simulink model "AcasXuClosedLoop_all_comp"  (Simulink 10.0)
settings: Solver=VariableStepAuto  StartTime=0.0  StopTime=20  RelTol=1e-3
libraries: neural x14040, simulink x2
(part 31 of 534)

== system AcasXuClosedLoop_all_comp/networks/Networks_m_7/AcasXu_4_7/Layer 5 ==
  "a{4} " : Inport  PortDimensions=50
      mask Display: e = 0.06;c1 = [120 230 180]/255;c2 = ([120 230 180]+0)/(256+128);c3 = ([120 230 180]+128)/(256+128);patch([0 1 1 0],[0 0 1 1],c1);patch([0 1 1 1-e 1-e 0+e],[0 0 1 1-e 0+e 0+e],c2);patch([0 0 1 1-e 0+e 0+e],[0 1 1 1-e 1-e 0+e],c3);plot([0 1 1 0 0],[0 0 1 1 0]);
  Delays 1 : Subsystem
      mask Display: e = 0.06;c1 = [120 150 230]/255;c2 = ([120 150 230]+0)/383;c3 = ([120 150 230]+128)/383;patch([0 1 1 0],[0 0 1 1],c1);patch([0 1 1 1-e 1-e 0+e],[0 0 1 1-e 0+e 0+e],c2);patch([0 0 1 1-e 0+e 0+e],[0 1 1 1-e 1-e 0+e],c3);plot([0 1 1 0 0],[0 0 1 1 0]);disp('0');
  "LW{5,4}" : Subsystem
      mask Display: e = 0.06;c1 = [120 150 230]/255;c2 = ([120 150 230]+0)/383;c3 = ([120 150 230]+128)/383;patch([0 1 1 0],[0 0 1 1],c1);patch([0 1 1 1-e 1-e 0+e],[0 0 1 1-e 0+e 0+e],c2);patch([0 0 1 1-e 0+e 0+e],[0 1 1 1-e 1-e 0+e],c3);plot([0 1 1 0 0],[0 0 1 1 0]);disp('W');
  "b{5}" : Constant  Value=[-1.29535000000000000142108547152020037174224853515625;4.1142500000000001847411112976260483264923095703125;0.037726299999999997336619372845234465785324573516845703125;-0.0252420999999999999541255846224885317496955394744873046875;-3.103639999999999954383156364201568067073822021484375;-0.0961853000000000013525180975193507038056850433349609375;2.682910000000000128039800983970053493976593017578125;2.18447000000000013386625141720287501811981201171875;-0.540381000000000000227373675443232059478759765625;-0.8092319999999999513562443098635412752628326416015625;5.54617999999999966576069709844887256622314453125;-1.3036700000000001065103560904390178620815277099609375;-0.173094999999999998863131622783839702606201171875;-1.215829999999999966320274324971251189708709716796875;0.5644869999999999610196255162009038031101226806640625;0.117124000000000005883293852093629539012908935546875;0.0181935000000000013375967000683885999023914337158203125;-2.12652000000000018786749933497048914432525634765625;-1.331609999999999960351715344586409628391265869140625;1.316429999999999989057641869294457137584686279296875;-0.919273000000000006792788553866557776927947998046875;-0.2169830000000000091997520712538971565663814544677734375;0.266203000000000022939872224014834500849246978759765625;3.121090000000000141966438604868017137050628662109375;-0.8987920000000000353423956767073832452297210693359375;-1.9803100000000000147082346302340738475322723388671875;-1.33654999999999990478727340814657509326934814453125;0.5149690000000000100754959930782206356525421142578125;-0.01195290000000000067703620487691296148113906383514404296875;-5.355109999999999814690454513765871524810791015625;1.3112399999999999611333123539225198328495025634765625;0.048415300000000001390265680356606026180088520050048828125;1.996750000000000024868995751603506505489349365234375;0.14216200000000001057998133546789176762104034423828125;-0.06109789999999999665636352119690855033695697784423828125;-2.012399999999999966604491419275291264057159423828125;1.7953900000000000414246414948138408362865447998046875;0.1728530000000000066417982225175364874303340911865234375;-0.411121000000000014207302001523203216493129730224609375;0.1690629999999999910631487409773399122059345245361328125;2.4055499999999998550492819049395620822906494140625;-0.06303730000000000444071446281668613664805889129638671875;1.6415100000000000246558329308754764497280120849609375;1.31810999999999989285015544737689197063446044921875;3.186749999999999971578290569595992565155029296875;2.004440000000000221547225009999237954616546630859375;-0.82457700000000000439825953435502015054225921630859375;-0.762615999999999960579089020029641687870025634765625;-1.0003800000000000469668748337426222860813140869140625;2.399080000000000101323394119390286505222320556640625]
      mask Display: e = 0.06;c1 = [120 150 230]/255;c2 = ([120 150 230]+0)/383;c3 = ([120 150 230]+128)/383;patch([0 1 1 0],[0 0 1 1],c1);patch([0 1 1 1-e 1-e 0+e],[0 0 1 1-e 0+e 0+e],c2);patch([0 0 1 1-e 0+e 0+e],[0 1 1 1-e 1-e 0+e],c3);plot([0 1 1 0 0],[0 0 1 1 0]);disp('b');
  netsum : Sum  Inputs=2  SaturateOnIntegerOverflow=on  InputSameDT=on  OutDataTypeStr=Inherit: Same as first input
      mask Display: e = 0.06;c1 = [120 150 230]/255;c2 = ([120 150 230]+0)/383;c3 = ([120 150 230]+128)/383;patch([0 1 1 0],[0 0 1 1],c1);patch([0 1 1 1-e 1-e 0+e],[0 0 1 1-e 0+e 0+e],c2);patch([0 0 1 1-e 0+e 0+e],[0 1 1 1-e 1-e 0+e],c3);plot([0 1 1 0 0],[0 0 1 1 0]);disp('+');
  poslin : neural/Transfer Functions/poslin
      settings: SourceProductName=Deep Learning Toolbox  SourceProductBaseCode=NN
  "a{5}" : Outport  InitialOutput=[0;0;0;0;0;0;0;0;0;0;0;0;0;0;0;0;0;0;0;0;0;0;0;0;0;0;0;0;0;0;0;0;0;0;0;0;0;0;0;0;0;0;0;0;0;0;0;0;0;0]
      mask Display: e = 0.06;c1 = [120 230 180]/255;c2 = ([120 230 180]+0)/(256+128);c3 = ([120 230 180]+128)/(256+128);patch([0 1 1 0],[0 0 1 1],c1);patch([0 1 1 1-e 1-e 0+e],[0 0 1 1-e 0+e 0+e],c2);patch([0 0 1 1-e 0+e 0+e],[0 1 1 1-e 1-e 0+e],c3);plot([0 1 1 0 0],[0 0 1 1 0]);
  wires:
  poslin/1 -> "a{5}"/1
  netsum/1 -> poslin/1
  "b{5}"/1 -> netsum/2
  "LW{5,4}"/1("lz{5,4}") -> netsum/1
  Delays 1/1("pd{5,4}") -> "LW{5,4}"/1("ad{5,4}")
  "a{4} "/1 -> Delays 1/1("p{5}")

== system AcasXuClosedLoop_all_comp/networks/Networks_m_7/AcasXu_4_7/Layer 6 ==
  "a{5} " : Inport  PortDimensions=50
      mask Display: e = 0.06;c1 = [120 230 180]/255;c2 = ([120 230 180]+0)/(256+128);c3 = ([120 230 180]+128)/(256+128);patch([0 1 1 0],[0 0 1 1],c1);patch([0 1 1 1-e 1-e 0+e],[0 0 1 1-e 0+e 0+e],c2);patch([0 0 1 1-e 0+e 0+e],[0 1 1 1-e 1-e 0+e],c3);plot([0 1 1 0 0],[0 0 1 1 0]);
  Delays 1 : Subsystem
      mask Display: e = 0.06;c1 = [120 150 230]/255;c2 = ([120 150 230]+0)/383;c3 = ([120 150 230]+128)/383;patch([0 1 1 0],[0 0 1 1],c1);patch([0 1 1 1-e 1-e 0+e],[0 0 1 1-e 0+e 0+e],c2);patch([0 0 1 1-e 0+e 0+e],[0 1 1 1-e 1-e 0+e],c3);plot([0 1 1 0 0],[0 0 1 1 0]);disp('0');
  "LW{6,5}" : Subsystem
      mask Display: e = 0.06;c1 = [120 150 230]/255;c2 = ([120 150 230]+0)/383;c3 = ([120 150 230]+128)/383;patch([0 1 1 0],[0 0 1 1],c1);patch([0 1 1 1-e 1-e 0+e],[0 0 1 1-e 0+e 0+e],c2);patch([0 0 1 1-e 0+e 0+e],[0 1 1 1-e 1-e 0+e],c3);plot([0 1 1 0 0],[0 0 1 1 0]);disp('W');
  "b{6}" : Constant  Value=[3.367739999999999955804241835721768438816070556640625;2.545879999999999920845539236324839293956756591796875;-0.0389668999999999987604581974665052257478237152099609375;-1.9147499999999999520383653361932374536991119384765625;-2.70427000000000017365664461976848542690277099609375;-3.99943999999999988403942552395164966583251953125;-0.4635569999999999968309793985099531710147857666015625;1.5156099999999999017319396443781442940235137939453125;0.5237119999999999553352836301201023161411285400390625;2.05891000000000001790567694115452468395233154296875;-4.25011999999999989796606314484961330890655517578125;0.775074000000000040699887904338538646697998046875;0.5163379999999999636628444932284764945507049560546875;-0.03237210000000000087450047203674330376088619232177734375;-3.05037000000000002586375558166764676570892333984375;-5.9134299999999999641886461176909506320953369140625;-0.05554019999999999790407656519164447672665119171142578125;7.9652300000000000324007487506605684757232666015625;-0.28509499999999998731681216668221168220043182373046875;4.55646000000000039875658330856822431087493896484375;-3.091439999999999965751840136363171041011810302734375;-0.01152899999999999931798999597276633721776306629180908203125;0.88857500000000000373034936274052597582340240478515625;1.3019700000000000716937620381941087543964385986328125;-1.7894799999999999595701183352502994239330291748046875;-2.40425000000000022026824808563105762004852294921875;-0.036211300000000001819699946281616576015949249267578125;-2.94575999999999993406163412146270275115966796875;-5.16894999999999971151964928139932453632354736328125;-4.540919999999999845385900698602199554443359375;0.95291599999999998527044908769312314689159393310546875;-0.0280836999999999996358024390019636484794318675994873046875;0.6533210000000000405862010666169226169586181640625;1.7611399999999999277378037731978110969066619873046875;-0.2219969999999999998863131622783839702606201171875;1.140579999999999927240423858165740966796875;-3.731050000000000199662508748588152229785919189453125;0.499856999999999995765165294869802892208099365234375;0.9889639999999999542268369623343460261821746826171875;-3.509069999999999911466375124291516840457916259765625;-3.913670000000000204209982257452793419361114501953125;-2.9911799999999999499777914024889469146728515625;4.86252999999999957481122692115604877471923828125;-2.406969999999999831885588719160296022891998291015625;0.58633900000000005459099838844849728047847747802734375;-0.031272000000000001185274101089817122556269168853759765625;-4.6410099999999996356336851022206246852874755859375;-5.66669999999999962625452099018730223178863525390625;-2.46628999999999987124965628026984632015228271484375;1.76560000000000005826450433232821524143218994140625]
      mask Display: e = 0.06;c1 = [120 150 230]/255;c2 = ([120 150 230]+0)/383;c3 = ([120 150 230]+128)/383;patch([0 1 1 0],[0 0 1 1],c1);patch([0 1 1 1-e 1-e 0+e],[0 0 1 1-e 0+e 0+e],c2);patch([0 0 1 1-e 0+e 0+e],[0 1 1 1-e 1-e 0+e],c3);plot([0 1 1 0 0],[0 0 1 1 0]);disp('b');
  netsum : Sum  Inputs=2  SaturateOnIntegerOverflow=on  InputSameDT=on  OutDataTypeStr=Inherit: Same as first input
      mask Display: e = 0.06;c1 = [120 150 230]/255;c2 = ([120 150 230]+0)/383;c3 = ([120 150 230]+128)/383;patch([0 1 1 0],[0 0 1 1],c1);patch([0 1 1 1-e 1-e 0+e],[0 0 1 1-e 0+e 0+e],c2);patch([0 0 1 1-e 0+e 0+e],[0 1 1 1-e 1-e 0+e],c3);plot([0 1 1 0 0],[0 0 1 1 0]);disp('+');
  poslin : neural/Transfer Functions/poslin
      settings: SourceProductName=Deep Learning Toolbox  SourceProductBaseCode=NN
  "a{6}" : Outport  InitialOutput=[0;0;0;0;0;0;0;0;0;0;0;0;0;0;0;0;0;0;0;0;0;0;0;0;0;0;0;0;0;0;0;0;0;0;0;0;0;0;0;0;0;0;0;0;0;0;0;0;0;0]
      mask Display: e = 0.06;c1 = [120 230 180]/255;c2 = ([120 230 180]+0)/(256+128);c3 = ([120 230 180]+128)/(256+128);patch([0 1 1 0],[0 0 1 1],c1);patch([0 1 1 1-e 1-e 0+e],[0 0 1 1-e 0+e 0+e],c2);patch([0 0 1 1-e 0+e 0+e],[0 1 1 1-e 1-e 0+e],c3);plot([0 1 1 0 0],[0 0 1 1 0]);
  wires:
  poslin/1 -> "a{6}"/1
  netsum/1 -> poslin/1
  "b{6}"/1 -> netsum/2
  "LW{6,5}"/1("lz{6,5}") -> netsum/1
  Delays 1/1("pd{6,5}") -> "LW{6,5}"/1("ad{6,5}")
  "a{5} "/1 -> Delays 1/1("p{6}")

== system AcasXuClosedLoop_all_comp/networks/Networks_m_7/AcasXu_4_7/Layer 7 ==
  "a{6} " : Inport  PortDimensions=50
      mask Display: e = 0.06;c1 = [120 230 180]/255;c2 = ([120 230 180]+0)/(256+128);c3 = ([120 230 180]+128)/(256+128);patch([0 1 1 0],[0 0 1 1],c1);patch([0 1 1 1-e 1-e 0+e],[0 0 1 1-e 0+e 0+e],c2);patch([0 0 1 1-e 0+e 0+e],[0 1 1 1-e 1-e 0+e],c3);plot([0 1 1 0 0],[0 0 1 1 0]);
  Delays 1 : Subsystem
      mask Display: e = 0.06;c1 = [120 150 230]/255;c2 = ([120 150 230]+0)/383;c3 = ([120 150 230]+128)/383;patch([0 1 1 0],[0 0 1 1],c1);patch([0 1 1 1-e 1-e 0+e],[0 0 1 1-e 0+e 0+e],c2);patch([0 0 1 1-e 0+e 0+e],[0 1 1 1-e 1-e 0+e],c3);plot([0 1 1 0 0],[0 0 1 1 0]);disp('0');
  "LW{7,6}" : Subsystem
      mask Display: e = 0.06;c1 = [120 150 230]/255;c2 = ([120 150 230]+0)/383;c3 = ([120 150 230]+128)/383;patch([0 1 1 0],[0 0 1 1],c1);patch([0 1 1 1-e 1-e 0+e],[0 0 1 1-e 0+e 0+e],c2);patch([0 0 1 1-e 0+e 0+e],[0 1 1 1-e 1-e 0+e],c3);plot([0 1 1 0 0],[0 0 1 1 0]);disp('W');
  "b{7}" : Constant  Value=[-0.0211303000000000011648904063576992484740912914276123046875;-0.0184695999999999992124966041728839627467095851898193359375;0.0182207999999999988027798991652161930687725543975830078125;-0.01842080000000000106208375427740975283086299896240234375;0.0182178999999999986780796490393186104483902454376220703125]
      mask Display: e = 0.06;c1 = [120 150 230]/255;c2 = ([120 150 230]+0)/383;c3 = ([120 150 230]+128)/383;patch([0 1 1 0],[0 0 1 1],c1);patch([0 1 1 1-e 1-e 0+e],[0 0 1 1-e 0+e 0+e],c2);patch([0 0 1 1-e 0+e 0+e],[0 1 1 1-e 1-e 0+e],c3);plot([0 1 1 0 0],[0 0 1 1 0]);disp('b');
  netsum : Sum  Inputs=2  SaturateOnIntegerOverflow=on  InputSameDT=on  OutDataTypeStr=Inherit: Same as first input
      mask Display: e = 0.06;c1 = [120 150 230]/255;c2 = ([120 150 230]+0)/383;c3 = ([120 150 230]+128)/383;patch([0 1 1 0],[0 0 1 1],c1);patch([0 1 1 1-e 1-e 0+e],[0 0 1 1-e 0+e 0+e],c2);patch([0 0 1 1-e 0+e 0+e],[0 1 1 1-e 1-e 0+e],c3);plot([0 1 1 0 0],[0 0 1 1 0]);disp('+');
  purelin : neural/Transfer Functions/purelin
      settings: SourceProductName=Deep Learning Toolbox
  "a{7}" : Outport  InitialOutput=[0;0;0;0;0]
      mask Display: e = 0.06;c1 = [120 230 180]/255;c2 = ([120 230 180]+0)/(256+128);c3 = ([120 230 180]+128)/(256+128);patch([0 1 1 0],[0 0 1 1],c1);patch([0 1 1 1-e 1-e 0+e],[0 0 1 1-e 0+e 0+e],c2);patch([0 0 1 1-e 0+e 0+e],[0 1 1 1-e 1-e 0+e],c3);plot([0 1 1 0 0],[0 0 1 1 0]);
  wires:
  purelin/1 -> "a{7}"/1
  netsum/1 -> purelin/1
  "b{7}"/1 -> netsum/2
  "LW{7,6}"/1("lz{7,6}") -> netsum/1
  Delays 1/1("pd{7,6}") -> "LW{7,6}"/1("ad{7,6}")
  "a{6} "/1 -> Delays 1/1("p{7}")

== system AcasXuClosedLoop_all_comp/networks/Networks_m_7/AcasXu_4_7/Process Input 1 ==
  x : Inport  PortDimensions=5
      mask Display: e = 0.06;c1 = [120 230 180]/255;c2 = ([120 230 180]+0)/(256+128);c3 = ([120 230 180]+128)/(256+128);patch([0 1 1 0],[0 0 1 1],c1);patch([0 1 1 1-e 1-e 0+e],[0 0 1 1-e 0+e 0+e],c2);patch([0 0 1 1-e 0+e 0+e],[0 1 1 1-e 1-e 0+e],c3);plot([0 1 1 0 0],[0 0 1 1 0]);
  p : Outport  PortDimensions=5
      mask Display: e = 0.06;c1 = [120 230 180]/255;c2 = ([120 230 180]+0)/(256+128);c3 = ([120 230 180]+128)/(256+128);patch([0 1 1 0],[0 0 1 1],c1);patch([0 1 1 1-e 1-e 0+e],[0 0 1 1-e 0+e 0+e],c2);patch([0 0 1 1-e 0+e 0+e],[0 1 1 1-e 1-e 0+e],c3);plot([0 1 1 0 0],[0 0 1 1 0]);
  wires:
  x/1 -> p/1

== system AcasXuClosedLoop_all_comp/networks/Networks_m_7/AcasXu_4_7/Process Output 1 ==
  a : Inport  PortDimensions=5
      mask Display: e = 0.06;c1 = [120 230 180]/255;c2 = ([120 230 180]+0)/(256+128);c3 = ([120 230 180]+128)/(256+128);patch([0 1 1 0],[0 0 1 1],c1);patch([0 1 1 1-e 1-e 0+e],[0 0 1 1-e 0+e 0+e],c2);patch([0 0 1 1-e 0+e 0+e],[0 1 1 1-e 1-e 0+e],c3);plot([0 1 1 0 0],[0 0 1 1 0]);
  y : Outport  PortDimensions=5
      mask Display: e = 0.06;c1 = [120 230 180]/255;c2 = ([120 230 180]+0)/(256+128);c3 = ([120 230 180]+128)/(256+128);patch([0 1 1 0],[0 0 1 1],c1);patch([0 1 1 1-e 1-e 0+e],[0 0 1 1-e 0+e 0+e],c2);patch([0 0 1 1-e 0+e 0+e],[0 1 1 1-e 1-e 0+e],c3);plot([0 1 1 0 0],[0 0 1 1 0]);
  wires:
  a/1 -> y/1

== system AcasXuClosedLoop_all_comp/networks/Networks_m_7/AcasXu_5_7/Layer 1 ==
  "p{1}" : Inport  PortDimensions=5
      mask Display: e = 0.06;c1 = [120 230 180]/255;c2 = ([120 230 180]+0)/(256+128);c3 = ([120 230 180]+128)/(256+128);patch([0 1 1 0],[0 0 1 1],c1);patch([0 1 1 1-e 1-e 0+e],[0 0 1 1-e 0+e 0+e],c2);patch([0 0 1 1-e 0+e 0+e],[0 1 1 1-e 1-e 0+e],c3);plot([0 1 1 0 0],[0 0 1 1 0]);
  Delays 1 : Subsystem
      mask Display: e = 0.06;c1 = [120 150 230]/255;c2 = ([120 150 230]+0)/383;c3 = ([120 150 230]+128)/383;patch([0 1 1 0],[0 0 1 1],c1);patch([0 1 1 1-e 1-e 0+e],[0 0 1 1-e 0+e 0+e],c2);patch([0 0 1 1-e 0+e 0+e],[0 1 1 1-e 1-e 0+e],c3);plot([0 1 1 0 0],[0 0 1 1 0]);disp('0');
  "IW{1,1}" : Subsystem
      mask Display: e = 0.06;c1 = [120 150 230]/255;c2 = ([120 150 230]+0)/383;c3 = ([120 150 230]+128)/383;patch([0 1 1 0],[0 0 1 1],c1);patch([0 1 1 1-e 1-e 0+e],[0 0 1 1-e 0+e 0+e],c2);patch([0 0 1 1-e 0+e 0+e],[0 1 1 1-e 1-e 0+e],c3);plot([0 1 1 0 0],[0 0 1 1 0]);disp('W');
  "b{1}" : Constant  Value=[0.025853700000000000291944246555431163869798183441162109375;-0.1536579999999999890381019440610543824732303619384765625;-0.09579020000000000589768234249277156777679920196533203125;0.01953850000000000031175062531474395655095577239990234375;-0.000513157999999999995220656412442394866957329213619232177734375;-0.256688000000000027256419343757443130016326904296875;-0.02045829999999999870841094207207788713276386260986328125;0.015036199999999999565858388450578786432743072509765625;-0.1940860000000000085140783312454004772007465362548828125;-0.6372079999999999966320274324971251189708709716796875;-0.398884999999999989572785352720529772341251373291015625;0.0055165999999999999758859559051415999419987201690673828125;-0.21925200000000000244426701101474463939666748046875;-0.322757000000000016104451106002670712769031524658203125;0.19237599999999999145217088880599476397037506103515625;-0.0266212000000000011123990617534218472428619861602783203125;-0.1476059999999999872155598268363974057137966156005859375;0.159337000000000006405542762877303175628185272216796875;0.371367999999999975901943116696202196180820465087890625;-0.67358499999999998930633182681049220263957977294921875;0.0638948000000000015941026276777847670018672943115234375;-0.14384099999999999663913285985472612082958221435546875;-0.2489180000000000003712585794346523471176624298095703125;-0.592006000000000032201796784647740423679351806640625;0.127653999999999989700683045157347805798053741455078125;-0.253425999999999984613197057115030474960803985595703125;-0.5876360000000000471942485091858543455600738525390625;0.029080000000000001680877659282487002201378345489501953125;0.291185000000000027142732506035827100276947021484375;-0.419733999999999995988986256634234450757503509521484375;-0.1534250000000000058175686490358202718198299407958984375;0.12184399999999999397726213601345079950988292694091796875;0.30686299999999999688071739001316018402576446533203125;-0.286256000000000010441425501994672231376171112060546875;0.08300100000000000532995869662045151926577091217041015625;0.06258149999999999824051855057405191473662853240966796875;-0.41020299999999998430411096705938689410686492919921875;0.08292960000000000608810779567647841759026050567626953125;-0.36475800000000002665245801836135797202587127685546875;-0.210280999999999995697663734972593374550342559814453125;0.0819209999999999938236072694053291343152523040771484375;-0.38086900000000001309530262005864642560482025146484375;-0.36825099999999999500488456760649569332599639892578125;-0.060613199999999999023625463223652332089841365814208984375;0.08224700000000000066346927951599354855716228485107421875;-0.1641789999999999916102666475126170553267002105712890625;-0.10791199999999999403765826855305931530892848968505859375;-0.274878000000000011215917083973181433975696563720703125;0.520432000000000005712763595511205494403839111328125;0.027086800000000001154010220716372714377939701080322265625]
      mask Display: e = 0.06;c1 = [120 150 230]/255;c2 = ([120 150 230]+0)/383;c3 = ([120 150 230]+128)/383;patch([0 1 1 0],[0 0 1 1],c1);patch([0 1 1 1-e 1-e 0+e],[0 0 1 1-e 0+e 0+e],c2);patch([0 0 1 1-e 0+e 0+e],[0 1 1 1-e 1-e 0+e],c3);plot([0 1 1 0 0],[0 0 1 1 0]);disp('b');
  netsum : Sum  Inputs=2  SaturateOnIntegerOverflow=on  InputSameDT=on  OutDataTypeStr=Inherit: Same as first input
      mask Display: e = 0.06;c1 = [120 150 230]/255;c2 = ([120 150 230]+0)/383;c3 = ([120 150 230]+128)/383;patch([0 1 1 0],[0 0 1 1],c1);patch([0 1 1 1-e 1-e 0+e],[0 0 1 1-e 0+e 0+e],c2);patch([0 0 1 1-e 0+e 0+e],[0 1 1 1-e 1-e 0+e],c3);plot([0 1 1 0 0],[0 0 1 1 0]);disp('+');
  poslin : neural/Transfer Functions/poslin
      settings: SourceProductName=Deep Learning Toolbox  SourceProductBaseCode=NN
  "a{1}" : Outport  InitialOutput=[0;0;0;0;0;0;0;0;0;0;0;0;0;0;0;0;0;0;0;0;0;0;0;0;0;0;0;0;0;0;0;0;0;0;0;0;0;0;0;0;0;0;0;0;0;0;0;0;0;0]
      mask Display: e = 0.06;c1 = [120 230 180]/255;c2 = ([120 230 180]+0)/(256+128);c3 = ([120 230 180]+128)/(256+128);patch([0 1 1 0],[0 0 1 1],c1);patch([0 1 1 1-e 1-e 0+e],[0 0 1 1-e 0+e 0+e],c2);patch([0 0 1 1-e 0+e 0+e],[0 1 1 1-e 1-e 0+e],c3);plot([0 1 1 0 0],[0 0 1 1 0]);
  wires:
  poslin/1 -> "a{1}"/1
  netsum/1 -> poslin/1
  "b{1}"/1 -> netsum/2
  "IW{1,1}"/1("iz{1,1}") -> netsum/1
  Delays 1/1("pd{1,1}") -> "IW{1,1}"/1("pd{1,1}")
  "p{1}"/1 -> Delays 1/1("p{1}")

== system AcasXuClosedLoop_all_comp/networks/Networks_m_7/AcasXu_5_7/Layer 2 ==
  "a{1} " : Inport  PortDimensions=50
      mask Display: e = 0.06;c1 = [120 230 180]/255;c2 = ([120 230 180]+0)/(256+128);c3 = ([120 230 180]+128)/(256+128);patch([0 1 1 0],[0 0 1 1],c1);patch([0 1 1 1-e 1-e 0+e],[0 0 1 1-e 0+e 0+e],c2);patch([0 0 1 1-e 0+e 0+e],[0 1 1 1-e 1-e 0+e],c3);plot([0 1 1 0 0],[0 0 1 1 0]);
  Delays 1 : Subsystem
      mask Display: e = 0.06;c1 = [120 150 230]/255;c2 = ([120 150 230]+0)/383;c3 = ([120 150 230]+128)/383;patch([0 1 1 0],[0 0 1 1],c1);patch([0 1 1 1-e 1-e 0+e],[0 0 1 1-e 0+e 0+e],c2);patch([0 0 1 1-e 0+e 0+e],[0 1 1 1-e 1-e 0+e],c3);plot([0 1 1 0 0],[0 0 1 1 0]);disp('0');
  "LW{2,1}" : Subsystem
      mask Display: e = 0.06;c1 = [120 150 230]/255;c2 = ([120 150 230]+0)/383;c3 = ([120 150 230]+128)/383;patch([0 1 1 0],[0 0 1 1],c1);patch([0 1 1 1-e 1-e 0+e],[0 0 1 1-e 0+e 0+e],c2);patch([0 0 1 1-e 0+e 0+e],[0 1 1 1-e 1-e 0+e],c3);plot([0 1 1 0 0],[0 0 1 1 0]);disp('W');
  "b{2}" : Constant  Value=[0.11085000000000000408562073062057606875896453857421875;-0.11289599999999999635491576555068604648113250732421875;-0.58576700000000003765165956792770884931087493896484375;-0.196506999999999987238652465748600661754608154296875;-0.0165311999999999993671284670426757656969130039215087890625;-0.039218099999999998794830702308900072239339351654052734375;-0.0174786000000000003862243858065994572825729846954345703125;-1.5495699999999998919264498908887617290019989013671875;0.135572999999999999065636302475468255579471588134765625;1.124940000000000051016968427575193345546722412109375;-0.0162874999999999998945288126606101286597549915313720703125;1.3120499999999999385380533567513339221477508544921875;1.809779999999999944293449516408145427703857421875;-0.8840179999999999704840547565254382789134979248046875;0.29213699999999998002664369778358377516269683837890625;-0.2073230000000000072812866847016266547143459320068359375;-0.0147159000000000005081712828314266516827046871185302734375;0.2425299999999999955857532540903775952756404876708984375;0.325168999999999985828225135264801792800426483154296875;0.4723910000000000053432813729159533977508544921875;0.804680999999999979621634338400326669216156005859375;-0.00365592999999999994364952016212555463425815105438232421875;-0.032890700000000001879119082559554954059422016143798828125;0.3019439999999999901802993917954154312610626220703125;0.90261599999999997390176531553152017295360565185546875;0.19002900000000000346744855050928890705108642578125;-0.047453900000000000247712961254364927299320697784423828125;-1.1688199999999999700861508244997821748256683349609375;-0.00785373999999999967969177561144533683545887470245361328125;-0.054647099999999997066435497572456370107829570770263671875;0.303854000000000012970957641300628893077373504638671875;-0.01024830000000000011783907183371411520056426525115966796875;-0.1605100000000000137756472895489423535764217376708984375;0.0523083000000000020168755554550443775951862335205078125;-0.3190589999999999815116780155221931636333465576171875;0.469619999999999981898923806511447764933109283447265625;0.2776680000000000259063881458132527768611907958984375;-0.86825399999999997024957565372460521757602691650390625;0.287171000000000009588774219082552008330821990966796875;1.2200699999999999878497192185022868216037750244140625;-0.09989620000000000443751702050576568581163883209228515625;0.84266300000000005088196530778077431023120880126953125;1.410579999999999945003992252168245613574981689453125;-0.0578737000000000001431743612556601874530315399169921875;-0.442159000000000024233060003098216839134693145751953125;-1.380290000000000016910917111090384423732757568359375;-0.56972100000000003294786665719584561884403228759765625;0.12313899999999999845812226340058259665966033935546875;0.63547600000000004083489102413295768201351165771484375;0.1860570000000000001616484723854227922856807708740234375]
      mask Display: e = 0.06;c1 = [120 150 230]/255;c2 = ([120 150 230]+0)/383;c3 = ([120 150 230]+128)/383;patch([0 1 1 0],[0 0 1 1],c1);patch([0 1 1 1-e 1-e 0+e],[0 0 1 1-e 0+e 0+e],c2);patch([0 0 1 1-e 0+e 0+e],[0 1 1 1-e 1-e 0+e],c3);plot([0 1 1 0 0],[0 0 1 1 0]);disp('b');
  netsum : Sum  Inputs=2  SaturateOnIntegerOverflow=on  InputSameDT=on  OutDataTypeStr=Inherit: Same as first input
      mask Display: e = 0.06;c1 = [120 150 230]/255;c2 = ([120 150 230]+0)/383;c3 = ([120 150 230]+128)/383;patch([0 1 1 0],[0 0 1 1],c1);patch([0 1 1 1-e 1-e 0+e],[0 0 1 1-e 0+e 0+e],c2);patch([0 0 1 1-e 0+e 0+e],[0 1 1 1-e 1-e 0+e],c3);plot([0 1 1 0 0],[0 0 1 1 0]);disp('+');
  poslin : neural/Transfer Functions/poslin
      settings: SourceProductName=Deep Learning Toolbox  SourceProductBaseCode=NN
  "a{2}" : Outport  InitialOutput=[0;0;0;0;0;0;0;0;0;0;0;0;0;0;0;0;0;0;0;0;0;0;0;0;0;0;0;0;0;0;0;0;0;0;0;0;0;0;0;0;0;0;0;0;0;0;0;0;0;0]
      mask Display: e = 0.06;c1 = [120 230 180]/255;c2 = ([120 230 180]+0)/(256+128);c3 = ([120 230 180]+128)/(256+128);patch([0 1 1 0],[0 0 1 1],c1);patch([0 1 1 1-e 1-e 0+e],[0 0 1 1-e 0+e 0+e],c2);patch([0 0 1 1-e 0+e 0+e],[0 1 1 1-e 1-e 0+e],c3);plot([0 1 1 0 0],[0 0 1 1 0]);
  wires:
  poslin/1 -> "a{2}"/1
  netsum/1 -> poslin/1
  "b{2}"/1 -> netsum/2
  "LW{2,1}"/1("lz{2,1}") -> netsum/1
  Delays 1/1("pd{2,1}") -> "LW{2,1}"/1("ad{2,1}")
  "a{1} "/1 -> Delays 1/1("p{2}")

== system AcasXuClosedLoop_all_comp/networks/Networks_m_7/AcasXu_5_7/Layer 3 ==
  "a{2} " : Inport  PortDimensions=50
      mask Display: e = 0.06;c1 = [120 230 180]/255;c2 = ([120 230 180]+0)/(256+128);c3 = ([120 230 180]+128)/(256+128);patch([0 1 1 0],[0 0 1 1],c1);patch([0 1 1 1-e 1-e 0+e],[0 0 1 1-e 0+e 0+e],c2);patch([0 0 1 1-e 0+e 0+e],[0 1 1 1-e 1-e 0+e],c3);plot([0 1 1 0 0],[0 0 1 1 0]);
  Delays 1 : Subsystem
      mask Display: e = 0.06;c1 = [120 150 230]/255;c2 = ([120 150 230]+0)/383;c3 = ([120 150 230]+128)/383;patch([0 1 1 0],[0 0 1 1],c1);patch([0 1 1 1-e 1-e 0+e],[0 0 1 1-e 0+e 0+e],c2);patch([0 0 1 1-e 0+e 0+e],[0 1 1 1-e 1-e 0+e],c3);plot([0 1 1 0 0],[0 0 1 1 0]);disp('0');
  "LW{3,2}" : Subsystem
      mask Display: e = 0.06;c1 = [120 150 230]/255;c2 = ([120 150 230]+0)/383;c3 = ([120 150 230]+128)/383;patch([0 1 1 0],[0 0 1 1],c1);patch([0 1 1 1-e 1-e 0+e],[0 0 1 1-e 0+e 0+e],c2);patch([0 0 1 1-e 0+e 0+e],[0 1 1 1-e 1-e 0+e],c3);plot([0 1 1 0 0],[0 0 1 1 0]);disp('W');
  "b{3}" : Constant  Value=[0.55666800000000005166356231711688451468944549560546875;-0.6085570000000000145945477925124578177928924560546875;-0.77388800000000002032862767009646631777286529541015625;-0.07211399999999999754773938320795423351228237152099609375;-0.433697999999999972420283711471711285412311553955078125;-0.25907299999999999773336867292528040707111358642578125;-0.480806000000000011151968237754772417247295379638671875;-1.286880000000000023874235921539366245269775390625;-0.54378300000000001634958834983990527689456939697265625;-0.493476999999999998980371174184256233274936676025390625;1.1728199999999999736388645033002831041812896728515625;0.1509979999999999933368854954096605069935321807861328125;-1.3989700000000000468247662865906022489070892333984375;-0.80389100000000002221867134721833281219005584716796875;-0.1987190000000000067448269192027510143816471099853515625;-0.2460130000000000094484420287699322216212749481201171875;-2.015070000000000138840050567523576319217681884765625;-0.2215209999999999956887819507755921222269535064697265625;0.399955999999999978200548866880126297473907470703125;-0.925227000000000021628920876537449657917022705078125;-0.02930169999999999996820321257473551668226718902587890625;-3.235370000000000079154460763675160706043243408203125;-1.1870600000000000040500935938325710594654083251953125;-0.149092000000000002302158463862724602222442626953125;-0.217602999999999990876631272840313613414764404296875;-1.3483400000000000940048039410612545907497406005859375;-3.215469999999999828332875040359795093536376953125;-0.382958999999999993857358049353933893144130706787109375;-2.703399999999999803179662194452248513698577880859375;-0.329566999999999998838262627032236196100711822509765625;1.8425700000000000411404244005098007619380950927734375;-0.882951999999999959101160129648633301258087158203125;-1.368209999999999926245664028101600706577301025390625;-0.254919999999999979944931283171172253787517547607421875;-1.4680999999999999605648781653144396841526031494140625;0.010692000000000000115019105351166217587888240814208984375;-0.455492999999999981231013634896953590214252471923828125;0.67115800000000003233679990444215945899486541748046875;-0.4913279999999999869686462261597625911235809326171875;-0.77535600000000004516920171226956881582736968994140625;-0.0429920000000000024353852268177433870732784271240234375;-0.01698020000000000084838802649755962193012237548828125;-2.859199999999999963762320476234890520572662353515625;-1.4817899999999999405275730168796144425868988037109375;-2.22435999999999989285015544737689197063446044921875;-2.1202100000000001500666257925331592559814453125;-0.10047899999999999887112522856114082969725131988525390625;-1.323329999999999895266000748961232602596282958984375;-0.5482110000000000038511416278197430074214935302734375;0.80128600000000005376676881496678106486797332763671875]
      mask Display: e = 0.06;c1 = [120 150 230]/255;c2 = ([120 150 230]+0)/383;c3 = ([120 150 230]+128)/383;patch([0 1 1 0],[0 0 1 1],c1);patch([0 1 1 1-e 1-e 0+e],[0 0 1 1-e 0+e 0+e],c2);patch([0 0 1 1-e 0+e 0+e],[0 1 1 1-e 1-e 0+e],c3);plot([0 1 1 0 0],[0 0 1 1 0]);disp('b');
  netsum : Sum  Inputs=2  SaturateOnIntegerOverflow=on  InputSameDT=on  OutDataTypeStr=Inherit: Same as first input
      mask Display: e = 0.06;c1 = [120 150 230]/255;c2 = ([120 150 230]+0)/383;c3 = ([120 150 230]+128)/383;patch([0 1 1 0],[0 0 1 1],c1);patch([0 1 1 1-e 1-e 0+e],[0 0 1 1-e 0+e 0+e],c2);patch([0 0 1 1-e 0+e 0+e],[0 1 1 1-e 1-e 0+e],c3);plot([0 1 1 0 0],[0 0 1 1 0]);disp('+');
  poslin : neural/Transfer Functions/poslin
      settings: SourceProductName=Deep Learning Toolbox  SourceProductBaseCode=NN
  "a{3}" : Outport  InitialOutput=[0;0;0;0;0;0;0;0;0;0;0;0;0;0;0;0;0;0;0;0;0;0;0;0;0;0;0;0;0;0;0;0;0;0;0;0;0;0;0;0;0;0;0;0;0;0;0;0;0;0]
      mask Display: e = 0.06;c1 = [120 230 180]/255;c2 = ([120 230 180]+0)/(256+128);c3 = ([120 230 180]+128)/(256+128);patch([0 1 1 0],[0 0 1 1],c1);patch([0 1 1 1-e 1-e 0+e],[0 0 1 1-e 0+e 0+e],c2);patch([0 0 1 1-e 0+e 0+e],[0 1 1 1-e 1-e 0+e],c3);plot([0 1 1 0 0],[0 0 1 1 0]);
  wires:
  poslin/1 -> "a{3}"/1
  netsum/1 -> poslin/1
  "b{3}"/1 -> netsum/2
  "LW{3,2}"/1("lz{3,2}") -> netsum/1
  Delays 1/1("pd{3,2}") -> "LW{3,2}"/1("ad{3,2}")
  "a{2} "/1 -> Delays 1/1("p{3}")

== system AcasXuClosedLoop_all_comp/networks/Networks_m_7/AcasXu_5_7/Layer 4 ==
  "a{3} " : Inport  PortDimensions=50
      mask Display: e = 0.06;c1 = [120 230 180]/255;c2 = ([120 230 180]+0)/(256+128);c3 = ([120 230 180]+128)/(256+128);patch([0 1 1 0],[0 0 1 1],c1);patch([0 1 1 1-e 1-e 0+e],[0 0 1 1-e 0+e 0+e],c2);patch([0 0 1 1-e 0+e 0+e],[0 1 1 1-e 1-e 0+e],c3);plot([0 1 1 0 0],[0 0 1 1 0]);
  Delays 1 : Subsystem
      mask Display: e = 0.06;c1 = [120 150 230]/255;c2 = ([120 150 230]+0)/383;c3 = ([120 150 230]+128)/383;patch([0 1 1 0],[0 0 1 1],c1);patch([0 1 1 1-e 1-e 0+e],[0 0 1 1-e 0+e 0+e],c2);patch([0 0 1 1-e 0+e 0+e],[0 1 1 1-e 1-e 0+e],c3);plot([0 1 1 0 0],[0 0 1 1 0]);disp('0');
  "LW{4,3}" : Subsystem
      mask Display: e = 0.06;c1 = [120 150 230]/255;c2 = ([120 150 230]+0)/383;c3 = ([120 150 230]+128)/383;patch([0 1 1 0],[0 0 1 1],c1);patch([0 1 1 1-e 1-e 0+e],[0 0 1 1-e 0+e 0+e],c2);patch([0 0 1 1-e 0+e 0+e],[0 1 1 1-e 1-e 0+e],c3);plot([0 1 1 0 0],[0 0 1 1 0]);disp('W');
  "b{4}" : Constant  Value=[-1.4390199999999999658228944099391810595989227294921875;-2.081139999999999989910293152206577360630035400390625;-1.3699399999999999355537738665589131414890289306640625;-1.1351999999999999868549593884381465613842010498046875;2.70664000000000015688783605583012104034423828125;1.9219299999999999162270114538841880857944488525390625;1.92982999999999993434585121576674282550811767578125;-4.06240999999999985448084771633148193359375;-1.4630799999999999361222080551669932901859283447265625;-0.09641379999999999395043914773850701749324798583984375;-2.02536000000000004916955731459893286228179931640625;-1.388640000000000096491703516221605241298675537109375;-0.75220100000000000850519654704839922487735748291015625;5.2182300000000001460875864722765982151031494140625;0.8931210000000000537312416781787760555744171142578125;-1.7022999999999999243271986415493302047252655029296875;1.658749999999999946709294817992486059665679931640625;-1.3010999999999999232613845379091799259185791015625;-2.519719999999999959783281155978329479694366455078125;-0.0312427000000000017199131008283075061626732349395751953125;1.5687100000000000488142859467188827693462371826171875;-0.427051000000000013923084907219163142144680023193359375;0.9480560000000000098197006082045845687389373779296875;-0.28132699999999999374011849795351736247539520263671875;1.1380399999999999405275730168796144425868988037109375;-0.63561199999999995480237657830002717673778533935546875;-1.6956700000000000105870867628254927694797515869140625;0.416663999999999978829379188027814961969852447509765625;0.6277559999999999806874484420404769480228424072265625;1.193410000000000081854523159563541412353515625;3.282830000000000136850530907395295798778533935546875;-2.248299999999999965183405947755090892314910888671875;-0.786456999999999961659113978384993970394134521484375;1.044470000000000009521272659185342490673065185546875;-0.118086999999999997523758565876050852239131927490234375;-0.10377699999999999425082108928108937107026576995849609375;-1.8642000000000000792255150372511707246303558349609375;-0.034299299999999997845545607333406223915517330169677734375;1.4412100000000001021049911287263967096805572509765625;-3.05060999999999982179588187136687338352203369140625;0.1675310000000000132391875240500667132437229156494140625;1.2728800000000000114397380457376129925251007080078125;-0.040699600000000002442135382807464338839054107666015625;-3.082640000000000046753712013014592230319976806640625;0.496636000000000021881163547732285223901271820068359375;-0.004864799999999999853550480821695600752718746662139892578125;-0.015449600000000000832311997100987355224788188934326171875;0.406996999999999997665867113028070889413356781005859375;3.099019999999999885886836636927910149097442626953125;-1.2769600000000000949995637711253948509693145751953125]
      mask Display: e = 0.06;c1 = [120 150 230]/255;c2 = ([120 150 230]+0)/383;c3 = ([120 150 230]+128)/383;patch([0 1 1 0],[0 0 1 1],c1);patch([0 1 1 1-e 1-e 0+e],[0 0 1 1-e 0+e 0+e],c2);patch([0 0 1 1-e 0+e 0+e],[0 1 1 1-e 1-e 0+e],c3);plot([0 1 1 0 0],[0 0 1 1 0]);disp('b');
  netsum : Sum  Inputs=2  SaturateOnIntegerOverflow=on  InputSameDT=on  OutDataTypeStr=Inherit: Same as first input
      mask Display: e = 0.06;c1 = [120 150 230]/255;c2 = ([120 150 230]+0)/383;c3 = ([120 150 230]+128)/383;patch([0 1 1 0],[0 0 1 1],c1);patch([0 1 1 1-e 1-e 0+e],[0 0 1 1-e 0+e 0+e],c2);patch([0 0 1 1-e 0+e 0+e],[0 1 1 1-e 1-e 0+e],c3);plot([0 1 1 0 0],[0 0 1 1 0]);disp('+');
  poslin : neural/Transfer Functions/poslin
      settings: SourceProductName=Deep Learning Toolbox  SourceProductBaseCode=NN
  "a{4}" : Outport  InitialOutput=[0;0;0;0;0;0;0;0;0;0;0;0;0;0;0;0;0;0;0;0;0;0;0;0;0;0;0;0;0;0;0;0;0;0;0;0;0;0;0;0;0;0;0;0;0;0;0;0;0;0]
      mask Display: e = 0.06;c1 = [120 230 180]/255;c2 = ([120 230 180]+0)/(256+128);c3 = ([120 230 180]+128)/(256+128);patch([0 1 1 0],[0 0 1 1],c1);patch([0 1 1 1-e 1-e 0+e],[0 0 1 1-e 0+e 0+e],c2);patch([0 0 1 1-e 0+e 0+e],[0 1 1 1-e 1-e 0+e],c3);plot([0 1 1 0 0],[0 0 1 1 0]);
  wires:
  poslin/1 -> "a{4}"/1
  netsum/1 -> poslin/1
  "b{4}"/1 -> netsum/2
  "LW{4,3}"/1("lz{4,3}") -> netsum/1
  Delays 1/1("pd{4,3}") -> "LW{4,3}"/1("ad{4,3}")
  "a{3} "/1 -> Delays 1/1("p{4}")

== system AcasXuClosedLoop_all_comp/networks/Networks_m_7/AcasXu_5_7/Layer 5 ==
  "a{4} " : Inport  PortDimensions=50
      mask Display: e = 0.06;c1 = [120 230 180]/255;c2 = ([120 230 180]+0)/(256+128);c3 = ([120 230 180]+128)/(256+128);patch([0 1 1 0],[0 0 1 1],c1);patch([0 1 1 1-e 1-e 0+e],[0 0 1 1-e 0+e 0+e],c2);patch([0 0 1 1-e 0+e 0+e],[0 1 1 1-e 1-e 0+e],c3);plot([0 1 1 0 0],[0 0 1 1 0]);
  Delays 1 : Subsystem
      mask Display: e = 0.06;c1 = [120 150 230]/255;c2 = ([120 150 230]+0)/383;c3 = ([120 150 230]+128)/383;patch([0 1 1 0],[0 0 1 1],c1);patch([0 1 1 1-e 1-e 0+e],[0 0 1 1-e 0+e 0+e],c2);patch([0 0 1 1-e 0+e 0+e],[0 1 1 1-e 1-e 0+e],c3);plot([0 1 1 0 0],[0 0 1 1 0]);disp('0');
  "LW{5,4}" : Subsystem
      mask Display: e = 0.06;c1 = [120 150 230]/255;c2 = ([120 150 230]+0)/383;c3 = ([120 150 230]+128)/383;patch([0 1 1 0],[0 0 1 1],c1);patch([0 1 1 1-e 1-e 0+e],[0 0 1 1-e 0+e 0+e],c2);patch([0 0 1 1-e 0+e 0+e],[0 1 1 1-e 1-e 0+e],c3);plot([0 1 1 0 0],[0 0 1 1 0]);disp('W');
  "b{5}" : Constant  Value=[-0.020954899999999998527755451505072414875030517578125;1.099369999999999958362195684458129107952117919921875;-0.348920999999999981167064788678544573485851287841796875;-4.51827999999999985192289386759512126445770263671875;1.0749999999999999555910790149937383830547332763671875;1.5686299999999999688071739001316018402576446533203125;-0.0092203200000000005986944273672634153626859188079833984375;-1.0860099999999999198507794062606990337371826171875;-1.1986399999999999277378037731978110969066619873046875;1.2360100000000000530775423612794838845729827880859375;1.5253099999999999436539610542240552604198455810546875;-0.6671359999999999512709791815723292529582977294921875;-3.484059999999999934772176857222802937030792236328125;0.486233999999999999541699935434735380113124847412109375;0.447741000000000000103028696685214526951313018798828125;-3.249420000000000197104554899851791560649871826171875;-2.567569999999999907913661445491015911102294921875;1.2615700000000000802202748673153109848499298095703125;-0.373989999999999989110932574476464651525020599365234375;-0.84059600000000000985522774499258957803249359130859375;-2.161979999999999790816218592226505279541015625;-0.03904359999999999775166514837110298685729503631591796875;-1.02141999999999999459987520822323858737945556640625;-5.76053000000000015035084288683719933032989501953125;-0.929035999999999972942532622255384922027587890625;-5.70530000000000026005864128819666802883148193359375;2.059219999999999828332875040359795093536376953125;1.8299600000000000310507175527163781225681304931640625;-0.9641539999999999555058138867025263607501983642578125;1.3219600000000000239452901951153762638568878173828125;-1.4874400000000000954258894125814549624919891357421875;0.7236920000000000019468870959826745092868804931640625;-2.977160000000000028563817977556027472019195556640625;-1.369169999999999998152588887023739516735076904296875;0.0313705999999999984861887014631065540015697479248046875;-1.0891200000000000880362449606764130294322967529296875;-0.2263030000000000041548986473571858368813991546630859375;2.316359999999999974562570059788413345813751220703125;0.9277450000000000418509671362699009478092193603515625;1.54797999999999991160848367144353687763214111328125;1.832829999999999959214846967370249330997467041015625;-1.9759800000000000697042423780658282339572906494140625;-0.6846520000000000383266751668998040258884429931640625;-2.063709999999999933351091385702602565288543701171875;-0.92198700000000000098765440270653925836086273193359375;-1.008459999999999912034809312899596989154815673828125;-2.846060000000000034248159863636828958988189697265625;-0.91065899999999999625543978254427202045917510986328125;1.803290000000000059543481256696395576000213623046875;0.11177800000000000235633734746443224139511585235595703125]
      mask Display: e = 0.06;c1 = [120 150 230]/255;c2 = ([120 150 230]+0)/383;c3 = ([120 150 230]+128)/383;patch([0 1 1 0],[0 0 1 1],c1);patch([0 1 1 1-e 1-e 0+e],[0 0 1 1-e 0+e 0+e],c2);patch([0 0 1 1-e 0+e 0+e],[0 1 1 1-e 1-e 0+e],c3);plot([0 1 1 0 0],[0 0 1 1 0]);disp('b');
  netsum : Sum  Inputs=2  SaturateOnIntegerOverflow=on  InputSameDT=on  OutDataTypeStr=Inherit: Same as first input
      mask Display: e = 0.06;c1 = [120 150 230]/255;c2 = ([120 150 230]+0)/383;c3 = ([120 150 230]+128)/383;patch([0 1 1 0],[0 0 1 1],c1);patch([0 1 1 1-e 1-e 0+e],[0 0 1 1-e 0+e 0+e],c2);patch([0 0 1 1-e 0+e 0+e],[0 1 1 1-e 1-e 0+e],c3);plot([0 1 1 0 0],[0 0 1 1 0]);disp('+');
  poslin : neural/Transfer Functions/poslin
      settings: SourceProductName=Deep Learning Toolbox  SourceProductBaseCode=NN
  "a{5}" : Outport  InitialOutput=[0;0;0;0;0;0;0;0;0;0;0;0;0;0;0;0;0;0;0;0;0;0;0;0;0;0;0;0;0;0;0;0;0;0;0;0;0;0;0;0;0;0;0;0;0;0;0;0;0;0]
      mask Display: e = 0.06;c1 = [120 230 180]/255;c2 = ([120 230 180]+0)/(256+128);c3 = ([120 230 180]+128)/(256+128);patch([0 1 1 0],[0 0 1 1],c1);patch([0 1 1 1-e 1-e 0+e],[0 0 1 1-e 0+e 0+e],c2);patch([0 0 1 1-e 0+e 0+e],[0 1 1 1-e 1-e 0+e],c3);plot([0 1 1 0 0],[0 0 1 1 0]);
  wires:
  poslin/1 -> "a{5}"/1
  netsum/1 -> poslin/1
  "b{5}"/1 -> netsum/2
  "LW{5,4}"/1("lz{5,4}") -> netsum/1
  Delays 1/1("pd{5,4}") -> "LW{5,4}"/1("ad{5,4}")
  "a{4} "/1 -> Delays 1/1("p{5}")

== system AcasXuClosedLoop_all_comp/networks/Networks_m_7/AcasXu_5_7/Layer 6 ==
  "a{5} " : Inport  PortDimensions=50
      mask Display: e = 0.06;c1 = [120 230 180]/255;c2 = ([120 230 180]+0)/(256+128);c3 = ([120 230 180]+128)/(256+128);patch([0 1 1 0],[0 0 1 1],c1);patch([0 1 1 1-e 1-e 0+e],[0 0 1 1-e 0+e 0+e],c2);patch([0 0 1 1-e 0+e 0+e],[0 1 1 1-e 1-e 0+e],c3);plot([0 1 1 0 0],[0 0 1 1 0]);
  Delays 1 : Subsystem
      mask Display: e = 0.06;c1 = [120 150 230]/255;c2 = ([120 150 230]+0)/383;c3 = ([120 150 230]+128)/383;patch([0 1 1 0],[0 0 1 1],c1);patch([0 1 1 1-e 1-e 0+e],[0 0 1 1-e 0+e 0+e],c2);patch([0 0 1 1-e 0+e 0+e],[0 1 1 1-e 1-e 0+e],c3);plot([0 1 1 0 0],[0 0 1 1 0]);disp('0');
  "LW{6,5}" : Subsystem
      mask Display: e = 0.06;c1 = [120 150 230]/255;c2 = ([120 150 230]+0)/383;c3 = ([120 150 230]+128)/383;patch([0 1 1 0],[0 0 1 1],c1);patch([0 1 1 1-e 1-e 0+e],[0 0 1 1-e 0+e 0+e],c2);patch([0 0 1 1-e 0+e 0+e],[0 1 1 1-e 1-e 0+e],c3);plot([0 1 1 0 0],[0 0 1 1 0]);disp('W');
  "b{6}" : Constant  Value=[0.344249000000000027199575924896635115146636962890625;-6.47055999999999986727061696001328527927398681640625;-9.2038799999999998391331246239133179187774658203125;1.4587499999999999911182158029987476766109466552734375;0.87765700000000002045652536253328435122966766357421875;-2.886249999999999982236431605997495353221893310546875;-7.26574999999999970867747833835892379283905029296875;0.91783099999999995244337469557649455964565277099609375;-6.777029999999999887450030655600130558013916015625;-0.632425000000000014921397450962103903293609619140625;1.639720000000000066364691519993357360363006591796875;3.157220000000000137418965096003375947475433349609375;1.8988899999999999668176542400033213198184967041015625;0.33594299999999999162270114538841880857944488525390625;0.5831910000000000149356083056773059070110321044921875;-7.45314999999999994173549566767178475856781005859375;-0.424254999999999993232080441885045729577541351318359375;0.9287710000000000132303057398530654609203338623046875;0.47467599999999998683364310636534355580806732177734375;-0.2070140000000000035651481766763026826083660125732421875;-8.35707000000000022055246517993509769439697265625;-1.53685999999999989285015544737689197063446044921875;-1.6284899999999999931077354631270281970500946044921875;1.6610799999999998899369302307604812085628509521484375;0.0871452000000000059909410765612847171723842620849609375;-5.0469699999999999562305674771778285503387451171875;-5.568889999999999673718775738961994647979736328125;2.4273199999999999221245161606930196285247802734375;-6.14135999999999970810904414975084364414215087890625;-7.05499999999999971578290569595992565155029296875;4.27414999999999967172925607883371412754058837890625;-0.77744500000000005268674385661142878234386444091796875;3.63511000000000006338041202980093657970428466796875;0.89910199999999995679189623842830769717693328857421875;-8.734719999999999373585524153895676136016845703125;-5.0541999999999998038902049302123486995697021484375;0.113978999999999996983746086698374710977077484130859375;-7.13295999999999974505726640927605330944061279296875;-2.094030000000000057980287238024175167083740234375;0.05307989999999999930491156874268199317157268524169921875;-0.038028499999999999803268480036422261036932468414306640625;1.282410000000000049880100050359033048152923583984375;6.86779000000000028336444302112795412540435791015625;2.650539999999999896118652031873352825641632080078125;-0.048799000000000002097433338121845736168324947357177734375;-4.52254999999999984794385454733856022357940673828125;0.79928699999999996972377402926213108003139495849609375;0.8179030000000000466542360300081782042980194091796875;-9.9243100000000001870148480520583689212799072265625;3.3845200000000001949729266925714910030364990234375]
      mask Display: e = 0.06;c1 = [120 150 230]/255;c2 = ([120 150 230]+0)/383;c3 = ([120 150 230]+128)/383;patch([0 1 1 0],[0 0 1 1],c1);patch([0 1 1 1-e 1-e 0+e],[0 0 1 1-e 0+e 0+e],c2);patch([0 0 1 1-e 0+e 0+e],[0 1 1 1-e 1-e 0+e],c3);plot([0 1 1 0 0],[0 0 1 1 0]);disp('b');
  netsum : Sum  Inputs=2  SaturateOnIntegerOverflow=on  InputSameDT=on  OutDataTypeStr=Inherit: Same as first input
      mask Display: e = 0.06;c1 = [120 150 230]/255;c2 = ([120 150 230]+0)/383;c3 = ([120 150 230]+128)/383;patch([0 1 1 0],[0 0 1 1],c1);patch([0 1 1 1-e 1-e 0+e],[0 0 1 1-e 0+e 0+e],c2);patch([0 0 1 1-e 0+e 0+e],[0 1 1 1-e 1-e 0+e],c3);plot([0 1 1 0 0],[0 0 1 1 0]);disp('+');
  poslin : neural/Transfer Functions/poslin
      settings: SourceProductName=Deep Learning Toolbox  SourceProductBaseCode=NN
  "a{6}" : Outport  InitialOutput=[0;0;0;0;0;0;0;0;0;0;0;0;0;0;0;0;0;0;0;0;0;0;0;0;0;0;0;0;0;0;0;0;0;0;0;0;0;0;0;0;0;0;0;0;0;0;0;0;0;0]
      mask Display: e = 0.06;c1 = [120 230 180]/255;c2 = ([120 230 180]+0)/(256+128);c3 = ([120 230 180]+128)/(256+128);patch([0 1 1 0],[0 0 1 1],c1);patch([0 1 1 1-e 1-e 0+e],[0 0 1 1-e 0+e 0+e],c2);patch([0 0 1 1-e 0+e 0+e],[0 1 1 1-e 1-e 0+e],c3);plot([0 1 1 0 0],[0 0 1 1 0]);
  wires:
  poslin/1 -> "a{6}"/1
  netsum/1 -> poslin/1
  "b{6}"/1 -> netsum/2
  "LW{6,5}"/1("lz{6,5}") -> netsum/1
  Delays 1/1("pd{6,5}") -> "LW{6,5}"/1("ad{6,5}")
  "a{5} "/1 -> Delays 1/1("p{6}")

== system AcasXuClosedLoop_all_comp/networks/Networks_m_7/AcasXu_5_7/Layer 7 ==
  "a{6} " : Inport  PortDimensions=50
      mask Display: e = 0.06;c1 = [120 230 180]/255;c2 = ([120 230 180]+0)/(256+128);c3 = ([120 230 180]+128)/(256+128);patch([0 1 1 0],[0 0 1 1],c1);patch([0 1 1 1-e 1-e 0+e],[0 0 1 1-e 0+e 0+e],c2);patch([0 0 1 1-e 0+e 0+e],[0 1 1 1-e 1-e 0+e],c3);plot([0 1 1 0 0],[0 0 1 1 0]);
  Delays 1 : Subsystem
      mask Display: e = 0.06;c1 = [120 150 230]/255;c2 = ([120 150 230]+0)/383;c3 = ([120 150 230]+128)/383;patch([0 1 1 0],[0 0 1 1],c1);patch([0 1 1 1-e 1-e 0+e],[0 0 1 1-e 0+e 0+e],c2);patch([0 0 1 1-e 0+e 0+e],[0 1 1 1-e 1-e 0+e],c3);plot([0 1 1 0 0],[0 0 1 1 0]);disp('0');
  "LW{7,6}" : Subsystem
      mask Display: e = 0.06;c1 = [120 150 230]/255;c2 = ([120 150 230]+0)/383;c3 = ([120 150 230]+128)/383;patch([0 1 1 0],[0 0 1 1],c1);patch([0 1 1 1-e 1-e 0+e],[0 0 1 1-e 0+e 0+e],c2);patch([0 0 1 1-e 0+e 0+e],[0 1 1 1-e 1-e 0+e],c3);plot([0 1 1 0 0],[0 0 1 1 0]);disp('W');
  "b{7}" : Constant  Value=[-0.020162300000000001054534237709958688355982303619384765625;0.018108800000000001284572448412291123531758785247802734375;-0.0186092999999999987592591565999100566841661930084228515625;0.0180950999999999993794741470765075064264237880706787109375;-0.0187709999999999994912958101167532731778919696807861328125]
      mask Display: e = 0.06;c1 = [120 150 230]/255;c2 = ([120 150 230]+0)/383;c3 = ([120 150 230]+128)/383;patch([0 1 1 0],[0 0 1 1],c1);patch([0 1 1 1-e 1-e 0+e],[0 0 1 1-e 0+e 0+e],c2);patch([0 0 1 1-e 0+e 0+e],[0 1 1 1-e 1-e 0+e],c3);plot([0 1 1 0 0],[0 0 1 1 0]);disp('b');
  netsum : Sum  Inputs=2  SaturateOnIntegerOverflow=on  InputSameDT=on  OutDataTypeStr=Inherit: Same as first input
      mask Display: e = 0.06;c1 = [120 150 230]/255;c2 = ([120 150 230]+0)/383;c3 = ([120 150 230]+128)/383;patch([0 1 1 0],[0 0 1 1],c1);patch([0 1 1 1-e 1-e 0+e],[0 0 1 1-e 0+e 0+e],c2);patch([0 0 1 1-e 0+e 0+e],[0 1 1 1-e 1-e 0+e],c3);plot([0 1 1 0 0],[0 0 1 1 0]);disp('+');
  purelin : neural/Transfer Functions/purelin
      settings: SourceProductName=Deep Learning Toolbox
  "a{7}" : Outport  InitialOutput=[0;0;0;0;0]
      mask Display: e = 0.06;c1 = [120 230 180]/255;c2 = ([120 230 180]+0)/(256+128);c3 = ([120 230 180]+128)/(256+128);patch([0 1 1 0],[0 0 1 1],c1);patch([0 1 1 1-e 1-e 0+e],[0 0 1 1-e 0+e 0+e],c2);patch([0 0 1 1-e 0+e 0+e],[0 1 1 1-e 1-e 0+e],c3);plot([0 1 1 0 0],[0 0 1 1 0]);
  wires:
  purelin/1 -> "a{7}"/1
  netsum/1 -> purelin/1
  "b{7}"/1 -> netsum/2
  "LW{7,6}"/1("lz{7,6}") -> netsum/1
  Delays 1/1("pd{7,6}") -> "LW{7,6}"/1("ad{7,6}")
  "a{6} "/1 -> Delays 1/1("p{7}")

== system AcasXuClosedLoop_all_comp/networks/Networks_m_7/AcasXu_5_7/Process Input 1 ==
  x : Inport  PortDimensions=5
      mask Display: e = 0.06;c1 = [120 230 180]/255;c2 = ([120 230 180]+0)/(256+128);c3 = ([120 230 180]+128)/(256+128);patch([0 1 1 0],[0 0 1 1],c1);patch([0 1 1 1-e 1-e 0+e],[0 0 1 1-e 0+e 0+e],c2);patch([0 0 1 1-e 0+e 0+e],[0 1 1 1-e 1-e 0+e],c3);plot([0 1 1 0 0],[0 0 1 1 0]);
  p : Outport  PortDimensions=5
      mask Display: e = 0.06;c1 = [120 230 180]/255;c2 = ([120 230 180]+0)/(256+128);c3 = ([120 230 180]+128)/(256+128);patch([0 1 1 0],[0 0 1 1],c1);patch([0 1 1 1-e 1-e 0+e],[0 0 1 1-e 0+e 0+e],c2);patch([0 0 1 1-e 0+e 0+e],[0 1 1 1-e 1-e 0+e],c3);plot([0 1 1 0 0],[0 0 1 1 0]);
  wires:
  x/1 -> p/1
